simulink model "All_64_Coils"  (R2021b) (continued)
== matlab function All_64_Coils/Coil-3/Outer_5_Coils/Square-10/Square - 10 == (continued)

F_xx_10 = vol_cy * Magentization* (dBxp_1_dx + dBxn_1_dx)/m0;
F_xz_10 = vol_cy * Magentization* (dBxp_1_dz + dBxn_1_dz)/m0;
F_yy_10 = vol_cy * Magentization* (dByp_1_dy + dByn_1_dy)/m0;
F_yz_10 = vol_cy * Magentization* (dByp_1_dz + dByn_1_dz)/m0;
end

== matlab function All_64_Coils/Coil-26/Outer_5_Coils/Square-9/Square - 9 ==
  data: x input; F_xx_9 output; F_xz_9 output; y input; z input; I input; F_yy_9 output; F_yz_9 output
function [F_xx_9, F_xz_9, F_yy_9, F_yz_9] = force(x, y, z, I)

g = 200e-6;     %Coil spacing
m0 = 4*pi*1e-7;
radius = 3e-3;%m
height = 1e-3;%m
Magentization = 1.45; %T
vol_cy = pi*radius^2*height; %If Object is Cylinder, vol in m^3
% vol_sp = (4/3)*(pi * radius^3); %If Object is Sphere, vol in m^3

k = 0.4e-3; %additional length added to wires, to get concentric coil
%--------%--------%--------%--------%--------%--------%--------%----------
% Line along y - axis
%-%--------%--------%--------%--------%--------%--------%--------%--------
y1_1 = -0.6e-3 - 8*k;
y2_1 = 0.6e-3 + 8*k;
a_xp_1 = 9*g;  
a_xn_1 = -9*g;

k_yp_1 = 1/(4*pi*((a_xp_1-x)^2+z^2));
k_1yp_1 = (y2_1-y)/((y2_1-y)^2+(a_xp_1-x)^2+z^2)^0.5;
k_2yp_1 = (y1_1-y)/((y1_1-y)^2+(a_xp_1-x)^2+z^2)^0.5;

k_1yp_3 = (y2_1-y)/((y2_1-y)^2+(a_xp_1-x)^2+z^2)^1.5;
k_2yp_3 = (y1_1-y)/((y1_1-y)^2+(a_xp_1-x)^2+z^2)^1.5;

k_yn_1 = 1/(4*pi*((a_xn_1-x)^2+z^2));
k_1yn_1 = (y2_1-y)/((y2_1-y)^2+(a_xn_1-x)^2+z^2)^0.5;
k_2yn_1 = (y1_1-y)/((y1_1-y)^2+(a_xn_1-x)^2+z^2)^0.5;

k_1yn_3 = (y2_1-y)/((y2_1-y)^2+(a_xn_1-x)^2+z^2)^1.5;
k_2yn_3 = (y1_1-y)/((y1_1-y)^2+(a_xn_1-x)^2+z^2)^1.5;

hyp_x_1 = -(z*k_yp_1*(a_xp_1-x))*I*m0;
hyp_x1_1 = (k_yp_1*(k_1yp_1 - k_2yp_1))*2*4*pi;
hyp_x2_1 = k_1yp_3 - k_2yp_3;
dBxp_1_dx = hyp_x_1*(hyp_x1_1 + hyp_x2_1);

hyp_z_1 = (k_yp_1)*I*m0;
hyp_z1_1 = (k_1yp_1 - k_2yp_1)*(-1);
hyp_z2_1 = (k_yp_1*(k_1yp_1 - k_2yp_1)*(z^2))*4*pi*2;
hyp_z3_1 = (k_1yp_3 - k_2yp_3)*(z^2);
dBxp_1_dz = hyp_z_1*(hyp_z1_1 + hyp_z2_1 + hyp_z3_1);

hyn_x_1 = -(z*k_yn_1*(a_xn_1-x))*I*m0*(-1);
hyn_x1_1 = (k_yn_1*(k_1yn_1 - k_2yn_1))*2*4*pi;
hyn_x2_1 = k_1yn_3 - k_2yn_3;
dBxn_1_dx = hyn_x_1*(hyn_x1_1 + hyn_x2_1);

hyn_z_1 = (k_yn_1)*I*m0*(-1);
hyn_z1_1 = (k_1yn_1 - k_2yn_1)*(-1);
hyn_z2_1 = (k_yn_1*(k_1yn_1 - k_2yn_1)*(z^2))*4*pi*2;
hyn_z3_1 = (k_1yn_3 - k_2yn_3)*(z^2);
dBxn_1_dz = hyn_z_1*(hyn_z1_1 + hyn_z2_1 + hyn_z3_1);

%--------%--------%--------%--------%--------%--------%--------%----------
% Line along x - axis
%-%--------%--------%--------%--------%a--------%--------%--------%--------
x1_1 = -0.6e-3 - 8*k;
x2_1 = 0.6e-3 + 8*k;
a_yp_1 = 9*g;          %Distance of coil from (0,0)
a_yn_1 = -9*g;

k_xp_1 = 1/(4*pi*((a_yp_1-y)^2+z^2));
k_1xp_1 = (x2_1-x)/((x2_1-x)^2+(a_yp_1-y)^2+z^2)^0.5;
k_2xp_1 = (x1_1-x)/((x1_1-x)^2+(a_yp_1-y)^2+z^2)^0.5;

k_xn_1 = 1/(4*pi*((a_yn_1-y)^2+z^2));
k_1xn_1 = (x2_1-x)/((x2_1-x)^2+(a_yn_1-y)^2+z^2)^0.5;
k_2xn_1 = (x1_1-x)/((x1_1-x)^2+(a_yn_1-y)^2+z^2)^0.5;

k_1xp_3 = (x2_1-x)/((x2_1-x)^2+(a_yp_1-y)^2+z^2)^1.5;
k_2xp_3 = (x1_1-x)/((x1_1-x)^2+(a_yp_1-y)^2+z^2)^1.5;

k_1xn_3 = (x2_1-x)/((x2_1-x)^2+(a_yn_1-y)^2+z^2)^1.5;
k_2xn_3 = (x1_1-x)/((x1_1-x)^2+(a_yn_1-y)^2+z^2)^1.5;

hxp_x_1 = -(z*k_xp_1*(a_yp_1-y))*I*m0;
hxp_x1_1 = (k_xp_1*(k_1xp_1 - k_2xp_1))*2*4*pi;
hxp_x2_1 = k_1xp_3 - k_2xp_3;
dByp_1_dy = hxp_x_1*(hxp_x1_1 + hxp_x2_1);

hxp_z_1 = (k_xp_1)*I*m0;
hxp_z1_1 = (k_1xp_1 - k_2xp_1)*(-1);
hxp_z2_1 = (k_xp_1*(k_1xp_1 - k_2xp_1)*(z^2))*4*pi*2;
hxp_z3_1 = (k_1xp_3 - k_2xp_3)*(z^2);
dByp_1_dz = hxp_z_1*(hxp_z1_1 + hxp_z2_1 + hxp_z3_1);

hxn_x_1 = -(z*k_xn_1*(a_yn_1-y))*I*m0*(-1);
hxn_x1_1 = (k_xn_1*(k_1xn_1 - k_2xn_1))*2*4*pi;
hxn_x2_1 = k_1xn_3 - k_2xn_3;
dByn_1_dy = hxn_x_1*(hxn_x1_1 + hxn_x2_1);

hxn_z_1 = (k_xn_1)*I*m0*(-1);
hxn_z1_1 = (k_1xn_1 - k_2xn_1)*(-1);
hxn_z2_1 = (k_xn_1*(k_1xn_1 - k_2xn_1)*(z^2))*4*pi*2;
hxn_z3_1 = (k_1xn_3 - k_2xn_3)*(z^2);
dByn_1_dz = hxn_z_1*(hxn_z1_1 + hxn_z2_1 + hxn_z3_1);

F_xx_9 = vol_cy * Magentization* (dBxp_1_dx + dBxn_1_dx)/m0;
F_xz_9 = vol_cy * Magentization* (dBxp_1_dz + dBxn_1_dz)/m0;
F_yy_9 = vol_cy * Magentization* (dByp_1_dy + dByn_1_dy)/m0;
F_yz_9 = vol_cy * Magentization* (dByp_1_dz + dByn_1_dz)/m0;
end

== matlab function All_64_Coils/Coil-27/Inner_5_coils/Square-1/Square - 1 ==
  data: x input; F_xx_1 output; F_xz_1 output; y input; z input; I input; F_yy_1 output; F_yz_1 output
function [F_xx_1, F_xz_1, F_yy_1, F_yz_1] = force(x, y, z, I)

g = 200e-6;     %Coil spacing
m0 = 4*pi*1e-7;
radius = 3e-3;%m
height = 1e-3;%m
Magentization = 1.45; %T
vol_cy = pi*radius^2*height; %If Object is Cylinder, vol in m^3
% vol_sp = (4/3)*(pi * radius^3); %If Object is Sphere, vol in m^3

k = 0.4e-3; %additional length added to wires, to get concentric coil
%--------%--------%--------%--------%--------%--------%--------%----------
% Line along y - axis
%-%--------%--------%--------%--------%--------%--------%--------%--------
y1_1 = -0.6e-3;
y2_1 = 0.6e-3;
a_xp_1 = g;  
a_xn_1 = -g;

k_yp_1 = 1/(4*pi*((a_xp_1-x)^2+z^2));
k_1yp_1 = (y2_1-y)/((y2_1-y)^2+(a_xp_1-x)^2+z^2)^0.5;
k_2yp_1 = (y1_1-y)/((y1_1-y)^2+(a_xp_1-x)^2+z^2)^0.5;

k_1yp_3 = (y2_1-y)/((y2_1-y)^2+(a_xp_1-x)^2+z^2)^1.5;
k_2yp_3 = (y1_1-y)/((y1_1-y)^2+(a_xp_1-x)^2+z^2)^1.5;

k_yn_1 = 1/(4*pi*((a_xn_1-x)^2+z^2));
k_1yn_1 = (y2_1-y)/((y2_1-y)^2+(a_xn_1-x)^2+z^2)^0.5;
k_2yn_1 = (y1_1-y)/((y1_1-y)^2+(a_xn_1-x)^2+z^2)^0.5;

k_1yn_3 = (y2_1-y)/((y2_1-y)^2+(a_xn_1-x)^2+z^2)^1.5;
k_2yn_3 = (y1_1-y)/((y1_1-y)^2+(a_xn_1-x)^2+z^2)^1.5;

hyp_x_1 = -(z*k_yp_1*(a_xp_1-x))*I*m0;
hyp_x1_1 = (k_yp_1*(k_1yp_1 - k_2yp_1))*2*4*pi;
hyp_x2_1 = k_1yp_3 - k_2yp_3;
dBxp_1_dx = hyp_x_1*(hyp_x1_1 + hyp_x2_1);

hyp_z_1 = (k_yp_1)*I*m0;
hyp_z1_1 = (k_1yp_1 - k_2yp_1)*(-1);
hyp_z2_1 = (k_yp_1*(k_1yp_1 - k_2yp_1)*(z^2))*4*pi*2;
hyp_z3_1 = (k_1yp_3 - k_2yp_3)*(z^2);
dBxp_1_dz = hyp_z_1*(hyp_z1_1 + hyp_z2_1 + hyp_z3_1);

hyn_x_1 = -(z*k_yn_1*(a_xn_1-x))*I*m0*(-1);
hyn_x1_1 = (k_yn_1*(k_1yn_1 - k_2yn_1))*2*4*pi;
hyn_x2_1 = k_1yn_3 - k_2yn_3;
dBxn_1_dx = hyn_x_1*(hyn_x1_1 + hyn_x2_1);

hyn_z_1 = (k_yn_1)*I*m0*(-1);
hyn_z1_1 = (k_1yn_1 - k_2yn_1)*(-1);
hyn_z2_1 = (k_yn_1*(k_1yn_1 - k_2yn_1)*(z^2))*4*pi*2;
hyn_z3_1 = (k_1yn_3 - k_2yn_3)*(z^2);
dBxn_1_dz = hyn_z_1*(hyn_z1_1 + hyn_z2_1 + hyn_z3_1);

%--------%--------%--------%--------%--------%--------%--------%----------
% Line along x - axis
%-%--------%--------%--------%--------%a--------%--------%--------%--------
x1_1 = -0.6e-3;
x2_1 = 0.6e-3;
a_yp_1 = g;          %Distance of coil from (0,0)
a_yn_1 = -g;

k_xp_1 = 1/(4*pi*((a_yp_1-y)^2+z^2));
k_1xp_1 = (x2_1-x)/((x2_1-x)^2+(a_yp_1-y)^2+z^2)^0.5;
k_2xp_1 = (x1_1-x)/((x1_1-x)^2+(a_yp_1-y)^2+z^2)^0.5;

k_xn_1 = 1/(4*pi*((a_yn_1-y)^2+z^2));
k_1xn_1 = (x2_1-x)/((x2_1-x)^2+(a_yn_1-y)^2+z^2)^0.5;
k_2xn_1 = (x1_1-x)/((x1_1-x)^2+(a_yn_1-y)^2+z^2)^0.5;

k_1xp_3 = (x2_1-x)/((x2_1-x)^2+(a_yp_1-y)^2+z^2)^1.5;
k_2xp_3 = (x1_1-x)/((x1_1-x)^2+(a_yp_1-y)^2+z^2)^1.5;

k_1xn_3 = (x2_1-x)/((x2_1-x)^2+(a_yn_1-y)^2+z^2)^1.5;
k_2xn_3 = (x1_1-x)/((x1_1-x)^2+(a_yn_1-y)^2+z^2)^1.5;

hxp_x_1 = -(z*k_xp_1*(a_yp_1-y))*I*m0;
hxp_x1_1 = (k_xp_1*(k_1xp_1 - k_2xp_1))*2*4*pi;
hxp_x2_1 = k_1xp_3 - k_2xp_3;
dByp_1_dy = hxp_x_1*(hxp_x1_1 + hxp_x2_1);

hxp_z_1 = (k_xp_1)*I*m0;
hxp_z1_1 = (k_1xp_1 - k_2xp_1)*(-1);
hxp_z2_1 = (k_xp_1*(k_1xp_1 - k_2xp_1)*(z^2))*4*pi*2;
hxp_z3_1 = (k_1xp_3 - k_2xp_3)*(z^2);
dByp_1_dz = hxp_z_1*(hxp_z1_1 + hxp_z2_1 + hxp_z3_1);

hxn_x_1 = -(z*k_xn_1*(a_yn_1-y))*I*m0*(-1);
hxn_x1_1 = (k_xn_1*(k_1xn_1 - k_2xn_1))*2*4*pi;
hxn_x2_1 = k_1xn_3 - k_2xn_3;
dByn_1_dy = hxn_x_1*(hxn_x1_1 + hxn_x2_1);

hxn_z_1 = (k_xn_1)*I*m0*(-1);
hxn_z1_1 = (k_1xn_1 - k_2xn_1)*(-1);
hxn_z2_1 = (k_xn_1*(k_1xn_1 - k_2xn_1)*(z^2))*4*pi*2;
hxn_z3_1 = (k_1xn_3 - k_2xn_3)*(z^2);
dByn_1_dz = hxn_z_1*(hxn_z1_1 + hxn_z2_1 + hxn_z3_1);

F_xx_1 = vol_cy * Magentization* (dBxp_1_dx + dBxn_1_dx)/m0;
F_xz_1 = vol_cy * Magentization* (dBxp_1_dz + dBxn_1_dz)/m0;
F_yy_1 = vol_cy * Magentization* (dByp_1_dy + dByn_1_dy)/m0;
F_yz_1 = vol_cy * Magentization* (dByp_1_dz + dByn_1_dz)/m0;
end

== matlab function All_64_Coils/Coil-27/Inner_5_coils/Square-2/Square - 2 ==
  data: x input; F_xx_2 output; F_xz_2 output; y input; z input; I input; F_yy_2 output; F_yz_2 output
function [F_xx_2, F_xz_2, F_yy_2, F_yz_2] = force(x, y, z, I)

g = 200e-6;     %Coil spacing
m0 = 4*pi*1e-7;
radius = 3e-3;%m
height = 1e-3;%m
Magentization = 1.45; %T
vol_cy = pi*radius^2*height; %If Object is Cylinder, vol in m^3
% vol_sp = (4/3)*(pi * radius^3); %If Object is Sphere, vol in m^3

k = 0.4e-3; %additional length added to wires, to get concentric coil
%--------%--------%--------%--------%--------%--------%--------%----------
% Line along y - axis
%-%--------%--------%--------%--------%--------%--------%--------%--------
y1_1 = -0.6e-3 - k;
y2_1 = 0.6e-3 + k;
a_xp_1 = 2*g;  
a_xn_1 = -2*g;

k_yp_1 = 1/(4*pi*((a_xp_1-x)^2+z^2));
k_1yp_1 = (y2_1-y)/((y2_1-y)^2+(a_xp_1-x)^2+z^2)^0.5;
k_2yp_1 = (y1_1-y)/((y1_1-y)^2+(a_xp_1-x)^2+z^2)^0.5;

k_1yp_3 = (y2_1-y)/((y2_1-y)^2+(a_xp_1-x)^2+z^2)^1.5;
k_2yp_3 = (y1_1-y)/((y1_1-y)^2+(a_xp_1-x)^2+z^2)^1.5;

k_yn_1 = 1/(4*pi*((a_xn_1-x)^2+z^2));
k_1yn_1 = (y2_1-y)/((y2_1-y)^2+(a_xn_1-x)^2+z^2)^0.5;
k_2yn_1 = (y1_1-y)/((y1_1-y)^2+(a_xn_1-x)^2+z^2)^0.5;

k_1yn_3 = (y2_1-y)/((y2_1-y)^2+(a_xn_1-x)^2+z^2)^1.5;
k_2yn_3 = (y1_1-y)/((y1_1-y)^2+(a_xn_1-x)^2+z^2)^1.5;

hyp_x_1 = -(z*k_yp_1*(a_xp_1-x))*I*m0;
hyp_x1_1 = (k_yp_1*(k_1yp_1 - k_2yp_1))*2*4*pi;
hyp_x2_1 = k_1yp_3 - k_2yp_3;
dBxp_1_dx = hyp_x_1*(hyp_x1_1 + hyp_x2_1);

hyp_z_1 = (k_yp_1)*I*m0;
hyp_z1_1 = (k_1yp_1 - k_2yp_1)*(-1);
hyp_z2_1 = (k_yp_1*(k_1yp_1 - k_2yp_1)*(z^2))*4*pi*2;
hyp_z3_1 = (k_1yp_3 - k_2yp_3)*(z^2);
dBxp_1_dz = hyp_z_1*(hyp_z1_1 + hyp_z2_1 + hyp_z3_1);

hyn_x_1 = -(z*k_yn_1*(a_xn_1-x))*I*m0*(-1);
hyn_x1_1 = (k_yn_1*(k_1yn_1 - k_2yn_1))*2*4*pi;
hyn_x2_1 = k_1yn_3 - k_2yn_3;
dBxn_1_dx = hyn_x_1*(hyn_x1_1 + hyn_x2_1);

hyn_z_1 = (k_yn_1)*I*m0*(-1);
hyn_z1_1 = (k_1yn_1 - k_2yn_1)*(-1);
hyn_z2_1 = (k_yn_1*(k_1yn_1 - k_2yn_1)*(z^2))*4*pi*2;
hyn_z3_1 = (k_1yn_3 - k_2yn_3)*(z^2);
dBxn_1_dz = hyn_z_1*(hyn_z1_1 + hyn_z2_1 + hyn_z3_1);

%--------%--------%--------%--------%--------%--------%--------%----------
% Line along x - axis
%-%--------%--------%--------%--------%a--------%--------%--------%--------
x1_1 = -0.6e-3 - k;
x2_1 = 0.6e-3 + k;
a_yp_1 = 2*g;          %Distance of coil from (0,0)
a_yn_1 = -2*g;

k_xp_1 = 1/(4*pi*((a_yp_1-y)^2+z^2));
k_1xp_1 = (x2_1-x)/((x2_1-x)^2+(a_yp_1-y)^2+z^2)^0.5;
k_2xp_1 = (x1_1-x)/((x1_1-x)^2+(a_yp_1-y)^2+z^2)^0.5;

k_xn_1 = 1/(4*pi*((a_yn_1-y)^2+z^2));
k_1xn_1 = (x2_1-x)/((x2_1-x)^2+(a_yn_1-y)^2+z^2)^0.5;
k_2xn_1 = (x1_1-x)/((x1_1-x)^2+(a_yn_1-y)^2+z^2)^0.5;

k_1xp_3 = (x2_1-x)/((x2_1-x)^2+(a_yp_1-y)^2+z^2)^1.5;
k_2xp_3 = (x1_1-x)/((x1_1-x)^2+(a_yp_1-y)^2+z^2)^1.5;

k_1xn_3 = (x2_1-x)/((x2_1-x)^2+(a_yn_1-y)^2+z^2)^1.5;
k_2xn_3 = (x1_1-x)/((x1_1-x)^2+(a_yn_1-y)^2+z^2)^1.5;

hxp_x_1 = -(z*k_xp_1*(a_yp_1-y))*I*m0;
hxp_x1_1 = (k_xp_1*(k_1xp_1 - k_2xp_1))*2*4*pi;
hxp_x2_1 = k_1xp_3 - k_2xp_3;
dByp_1_dy = hxp_x_1*(hxp_x1_1 + hxp_x2_1);

hxp_z_1 = (k_xp_1)*I*m0;
hxp_z1_1 = (k_1xp_1 - k_2xp_1)*(-1);
hxp_z2_1 = (k_xp_1*(k_1xp_1 - k_2xp_1)*(z^2))*4*pi*2;
hxp_z3_1 = (k_1xp_3 - k_2xp_3)*(z^2);
dByp_1_dz = hxp_z_1*(hxp_z1_1 + hxp_z2_1 + hxp_z3_1);

hxn_x_1 = -(z*k_xn_1*(a_yn_1-y))*I*m0*(-1);
hxn_x1_1 = (k_xn_1*(k_1xn_1 - k_2xn_1))*2*4*pi;
hxn_x2_1 = k_1xn_3 - k_2xn_3;
dByn_1_dy = hxn_x_1*(hxn_x1_1 + hxn_x2_1);

hxn_z_1 = (k_xn_1)*I*m0*(-1);
hxn_z1_1 = (k_1xn_1 - k_2xn_1)*(-1);
hxn_z2_1 = (k_xn_1*(k_1xn_1 - k_2xn_1)*(z^2))*4*pi*2;
hxn_z3_1 = (k_1xn_3 - k_2xn_3)*(z^2);
dByn_1_dz = hxn_z_1*(hxn_z1_1 + hxn_z2_1 + hxn_z3_1);

F_xx_2 = vol_cy * Magentization* (dBxp_1_dx + dBxn_1_dx)/m0;
F_xz_2 = vol_cy * Magentization* (dBxp_1_dz + dBxn_1_dz)/m0;
F_yy_2 = vol_cy * Magentization* (dByp_1_dy + dByn_1_dy)/m0;
F_yz_2 = vol_cy * Magentization* (dByp_1_dz + dByn_1_dz)/m0;
end

== matlab function All_64_Coils/Coil-27/Inner_5_coils/Square-3/Square - 3 ==
  data: x input; F_xx_3 output; F_xz_3 output; y input; z input; I input; F_yy_3 output; F_yz_3 output
function [F_xx_3, F_xz_3, F_yy_3, F_yz_3] = force(x, y, z, I)

g = 200e-6;     %Coil spacing
m0 = 4*pi*1e-7;
radius = 3e-3;%m
height = 1e-3;%m
Magentization = 1.45; %T
vol_cy = pi*radius^2*height; %If Object is Cylinder, vol in m^3
% vol_sp = (4/3)*(pi * radius^3); %If Object is Sphere, vol in m^3

k = 0.4e-3; %additional length added to wires, to get concentric coil
%--------%--------%--------%--------%--------%--------%--------%----------
% Line along y - axis
%-%--------%--------%--------%--------%--------%--------%--------%--------
y1_1 = -0.6e-3 - 2*k;
y2_1 = 0.6e-3 + 2*k;
a_xp_1 = 3*g;  
a_xn_1 = -3*g;

k_yp_1 = 1/(4*pi*((a_xp_1-x)^2+z^2));
k_1yp_1 = (y2_1-y)/((y2_1-y)^2+(a_xp_1-x)^2+z^2)^0.5;
k_2yp_1 = (y1_1-y)/((y1_1-y)^2+(a_xp_1-x)^2+z^2)^0.5;

k_1yp_3 = (y2_1-y)/((y2_1-y)^2+(a_xp_1-x)^2+z^2)^1.5;
k_2yp_3 = (y1_1-y)/((y1_1-y)^2+(a_xp_1-x)^2+z^2)^1.5;

k_yn_1 = 1/(4*pi*((a_xn_1-x)^2+z^2));
k_1yn_1 = (y2_1-y)/((y2_1-y)^2+(a_xn_1-x)^2+z^2)^0.5;
k_2yn_1 = (y1_1-y)/((y1_1-y)^2+(a_xn_1-x)^2+z^2)^0.5;

k_1yn_3 = (y2_1-y)/((y2_1-y)^2+(a_xn_1-x)^2+z^2)^1.5;
k_2yn_3 = (y1_1-y)/((y1_1-y)^2+(a_xn_1-x)^2+z^2)^1.5;

hyp_x_1 = -(z*k_yp_1*(a_xp_1-x))*I*m0;
hyp_x1_1 = (k_yp_1*(k_1yp_1 - k_2yp_1))*2*4*pi;
hyp_x2_1 = k_1yp_3 - k_2yp_3;
dBxp_1_dx = hyp_x_1*(hyp_x1_1 + hyp_x2_1);

hyp_z_1 = (k_yp_1)*I*m0;
hyp_z1_1 = (k_1yp_1 - k_2yp_1)*(-1);
hyp_z2_1 = (k_yp_1*(k_1yp_1 - k_2yp_1)*(z^2))*4*pi*2;
hyp_z3_1 = (k_1yp_3 - k_2yp_3)*(z^2);
dBxp_1_dz = hyp_z_1*(hyp_z1_1 + hyp_z2_1 + hyp_z3_1);

hyn_x_1 = -(z*k_yn_1*(a_xn_1-x))*I*m0*(-1);
hyn_x1_1 = (k_yn_1*(k_1yn_1 - k_2yn_1))*2*4*pi;
hyn_x2_1 = k_1yn_3 - k_2yn_3;
dBxn_1_dx = hyn_x_1*(hyn_x1_1 + hyn_x2_1);

hyn_z_1 = (k_yn_1)*I*m0*(-1);
hyn_z1_1 = (k_1yn_1 - k_2yn_1)*(-1);
hyn_z2_1 = (k_yn_1*(k_1yn_1 - k_2yn_1)*(z^2))*4*pi*2;
hyn_z3_1 = (k_1yn_3 - k_2yn_3)*(z^2);
dBxn_1_dz = hyn_z_1*(hyn_z1_1 + hyn_z2_1 + hyn_z3_1);

%--------%--------%--------%--------%--------%--------%--------%----------
% Line along x - axis
%-%--------%--------%--------%--------%a--------%--------%--------%--------
x1_1 = -0.6e-3 - 2*k;
x2_1 = 0.6e-3 + 2*k;
a_yp_1 = 3*g;          %Distance of coil from (0,0)
a_yn_1 = -3*g;

k_xp_1 = 1/(4*pi*((a_yp_1-y)^2+z^2));
k_1xp_1 = (x2_1-x)/((x2_1-x)^2+(a_yp_1-y)^2+z^2)^0.5;
k_2xp_1 = (x1_1-x)/((x1_1-x)^2+(a_yp_1-y)^2+z^2)^0.5;

k_xn_1 = 1/(4*pi*((a_yn_1-y)^2+z^2));
k_1xn_1 = (x2_1-x)/((x2_1-x)^2+(a_yn_1-y)^2+z^2)^0.5;
k_2xn_1 = (x1_1-x)/((x1_1-x)^2+(a_yn_1-y)^2+z^2)^0.5;

k_1xp_3 = (x2_1-x)/((x2_1-x)^2+(a_yp_1-y)^2+z^2)^1.5;
k_2xp_3 = (x1_1-x)/((x1_1-x)^2+(a_yp_1-y)^2+z^2)^1.5;

k_1xn_3 = (x2_1-x)/((x2_1-x)^2+(a_yn_1-y)^2+z^2)^1.5;
k_2xn_3 = (x1_1-x)/((x1_1-x)^2+(a_yn_1-y)^2+z^2)^1.5;

hxp_x_1 = -(z*k_xp_1*(a_yp_1-y))*I*m0;
hxp_x1_1 = (k_xp_1*(k_1xp_1 - k_2xp_1))*2*4*pi;
hxp_x2_1 = k_1xp_3 - k_2xp_3;
dByp_1_dy = hxp_x_1*(hxp_x1_1 + hxp_x2_1);

hxp_z_1 = (k_xp_1)*I*m0;
hxp_z1_1 = (k_1xp_1 - k_2xp_1)*(-1);
hxp_z2_1 = (k_xp_1*(k_1xp_1 - k_2xp_1)*(z^2))*4*pi*2;
hxp_z3_1 = (k_1xp_3 - k_2xp_3)*(z^2);
dByp_1_dz = hxp_z_1*(hxp_z1_1 + hxp_z2_1 + hxp_z3_1);

hxn_x_1 = -(z*k_xn_1*(a_yn_1-y))*I*m0*(-1);
hxn_x1_1 = (k_xn_1*(k_1xn_1 - k_2xn_1))*2*4*pi;
hxn_x2_1 = k_1xn_3 - k_2xn_3;
dByn_1_dy = hxn_x_1*(hxn_x1_1 + hxn_x2_1);

hxn_z_1 = (k_xn_1)*I*m0*(-1);
hxn_z1_1 = (k_1xn_1 - k_2xn_1)*(-1);
hxn_z2_1 = (k_xn_1*(k_1xn_1 - k_2xn_1)*(z^2))*4*pi*2;
hxn_z3_1 = (k_1xn_3 - k_2xn_3)*(z^2);
dByn_1_dz = hxn_z_1*(hxn_z1_1 + hxn_z2_1 + hxn_z3_1);

F_xx_3 = vol_cy * Magentization* (dBxp_1_dx + dBxn_1_dx)/m0;
F_xz_3 = vol_cy * Magentization* (dBxp_1_dz + dBxn_1_dz)/m0;
F_yy_3 = vol_cy * Magentization* (dByp_1_dy + dByn_1_dy)/m0;
F_yz_3 = vol_cy * Magentization* (dByp_1_dz + dByn_1_dz)/m0;
end

== matlab function All_64_Coils/Coil-27/Inner_5_coils/Square-4/Square - 4 ==
  data: x input; F_xx_4 output; F_xz_4 output; y input; z input; I input; F_yy_4 output; F_yz_4 output
function [F_xx_4, F_xz_4, F_yy_4, F_yz_4] = force(x, y, z, I)

g = 200e-6;     %Coil spacing
m0 = 4*pi*1e-7;
radius = 3e-3;%m
height = 1e-3;%m
Magentization = 1.45; %T
vol_cy = pi*radius^2*height; %If Object is Cylinder, vol in m^3
% vol_sp = (4/3)*(pi * radius^3); %If Object is Sphere, vol in m^3

k = 0.4e-3; %additional length added to wires, to get concentric coil
%--------%--------%--------%--------%--------%--------%--------%----------
% Line along y - axis
%-%--------%--------%--------%--------%--------%--------%--------%--------
y1_1 = -0.6e-3 - 3*k;
y2_1 = 0.6e-3 + 3*k;
a_xp_1 = 4*g;  
a_xn_1 = -4*g;

k_yp_1 = 1/(4*pi*((a_xp_1-x)^2+z^2));
k_1yp_1 = (y2_1-y)/((y2_1-y)^2+(a_xp_1-x)^2+z^2)^0.5;
k_2yp_1 = (y1_1-y)/((y1_1-y)^2+(a_xp_1-x)^2+z^2)^0.5;

k_1yp_3 = (y2_1-y)/((y2_1-y)^2+(a_xp_1-x)^2+z^2)^1.5;
k_2yp_3 = (y1_1-y)/((y1_1-y)^2+(a_xp_1-x)^2+z^2)^1.5;

k_yn_1 = 1/(4*pi*((a_xn_1-x)^2+z^2));
k_1yn_1 = (y2_1-y)/((y2_1-y)^2+(a_xn_1-x)^2+z^2)^0.5;
k_2yn_1 = (y1_1-y)/((y1_1-y)^2+(a_xn_1-x)^2+z^2)^0.5;

k_1yn_3 = (y2_1-y)/((y2_1-y)^2+(a_xn_1-x)^2+z^2)^1.5;
k_2yn_3 = (y1_1-y)/((y1_1-y)^2+(a_xn_1-x)^2+z^2)^1.5;

hyp_x_1 = -(z*k_yp_1*(a_xp_1-x))*I*m0;
hyp_x1_1 = (k_yp_1*(k_1yp_1 - k_2yp_1))*2*4*pi;
hyp_x2_1 = k_1yp_3 - k_2yp_3;
dBxp_1_dx = hyp_x_1*(hyp_x1_1 + hyp_x2_1);

hyp_z_1 = (k_yp_1)*I*m0;
hyp_z1_1 = (k_1yp_1 - k_2yp_1)*(-1);
hyp_z2_1 = (k_yp_1*(k_1yp_1 - k_2yp_1)*(z^2))*4*pi*2;
hyp_z3_1 = (k_1yp_3 - k_2yp_3)*(z^2);
dBxp_1_dz = hyp_z_1*(hyp_z1_1 + hyp_z2_1 + hyp_z3_1);

hyn_x_1 = -(z*k_yn_1*(a_xn_1-x))*I*m0*(-1);
hyn_x1_1 = (k_yn_1*(k_1yn_1 - k_2yn_1))*2*4*pi;
hyn_x2_1 = k_1yn_3 - k_2yn_3;
dBxn_1_dx = hyn_x_1*(hyn_x1_1 + hyn_x2_1);

hyn_z_1 = (k_yn_1)*I*m0*(-1);
hyn_z1_1 = (k_1yn_1 - k_2yn_1)*(-1);
hyn_z2_1 = (k_yn_1*(k_1yn_1 - k_2yn_1)*(z^2))*4*pi*2;
hyn_z3_1 = (k_1yn_3 - k_2yn_3)*(z^2);
dBxn_1_dz = hyn_z_1*(hyn_z1_1 + hyn_z2_1 + hyn_z3_1);

%--------%--------%--------%--------%--------%--------%--------%----------
% Line along x - axis
%-%--------%--------%--------%--------%a--------%--------%--------%--------
x1_1 = -0.6e-3 - 3*k;
x2_1 = 0.6e-3 + 3*k;
a_yp_1 = 4*g;          %Distance of coil from (0,0)
a_yn_1 = -4*g;

k_xp_1 = 1/(4*pi*((a_yp_1-y)^2+z^2));
k_1xp_1 = (x2_1-x)/((x2_1-x)^2+(a_yp_1-y)^2+z^2)^0.5;
k_2xp_1 = (x1_1-x)/((x1_1-x)^2+(a_yp_1-y)^2+z^2)^0.5;

k_xn_1 = 1/(4*pi*((a_yn_1-y)^2+z^2));
k_1xn_1 = (x2_1-x)/((x2_1-x)^2+(a_yn_1-y)^2+z^2)^0.5;
k_2xn_1 = (x1_1-x)/((x1_1-x)^2+(a_yn_1-y)^2+z^2)^0.5;

k_1xp_3 = (x2_1-x)/((x2_1-x)^2+(a_yp_1-y)^2+z^2)^1.5;
k_2xp_3 = (x1_1-x)/((x1_1-x)^2+(a_yp_1-y)^2+z^2)^1.5;

k_1xn_3 = (x2_1-x)/((x2_1-x)^2+(a_yn_1-y)^2+z^2)^1.5;
k_2xn_3 = (x1_1-x)/((x1_1-x)^2+(a_yn_1-y)^2+z^2)^1.5;

hxp_x_1 = -(z*k_xp_1*(a_yp_1-y))*I*m0;
hxp_x1_1 = (k_xp_1*(k_1xp_1 - k_2xp_1))*2*4*pi;
hxp_x2_1 = k_1xp_3 - k_2xp_3;
dByp_1_dy = hxp_x_1*(hxp_x1_1 + hxp_x2_1);

hxp_z_1 = (k_xp_1)*I*m0;
hxp_z1_1 = (k_1xp_1 - k_2xp_1)*(-1);
hxp_z2_1 = (k_xp_1*(k_1xp_1 - k_2xp_1)*(z^2))*4*pi*2;
hxp_z3_1 = (k_1xp_3 - k_2xp_3)*(z^2);
dByp_1_dz = hxp_z_1*(hxp_z1_1 + hxp_z2_1 + hxp_z3_1);

hxn_x_1 = -(z*k_xn_1*(a_yn_1-y))*I*m0*(-1);
hxn_x1_1 = (k_xn_1*(k_1xn_1 - k_2xn_1))*2*4*pi;
hxn_x2_1 = k_1xn_3 - k_2xn_3;
dByn_1_dy = hxn_x_1*(hxn_x1_1 + hxn_x2_1);

hxn_z_1 = (k_xn_1)*I*m0*(-1);
hxn_z1_1 = (k_1xn_1 - k_2xn_1)*(-1);
hxn_z2_1 = (k_xn_1*(k_1xn_1 - k_2xn_1)*(z^2))*4*pi*2;
hxn_z3_1 = (k_1xn_3 - k_2xn_3)*(z^2);
dByn_1_dz = hxn_z_1*(hxn_z1_1 + hxn_z2_1 + hxn_z3_1);

F_xx_4 = vol_cy * Magentization* (dBxp_1_dx + dBxn_1_dx)/m0;
F_xz_4 = vol_cy * Magentization* (dBxp_1_dz + dBxn_1_dz)/m0;
F_yy_4 = vol_cy * Magentization* (dByp_1_dy + dByn_1_dy)/m0;
F_yz_4 = vol_cy * Magentization* (dByp_1_dz + dByn_1_dz)/m0;
end

== matlab function All_64_Coils/Coil-27/Inner_5_coils/Square-5/Square - 5 ==
  data: x input; F_xx_5 output; F_xz_5 output; y input; z input; I input; F_yy_5 output; F_yz_5 output
function [F_xx_5, F_xz_5, F_yy_5, F_yz_5] = force(x, y, z, I)

g = 200e-6;     %Coil spacing
m0 = 4*pi*1e-7;
radius = 3e-3;%m
height = 1e-3;%m
Magentization = 1.45; %T
vol_cy = pi*radius^2*height; %If Object is Cylinder, vol in m^3
% vol_sp = (4/3)*(pi * radius^3); %If Object is Sphere, vol in m^3

k = 0.4e-3; %additional length added to wires, to get concentric coil
%--------%--------%--------%--------%--------%--------%--------%----------
% Line along y - axis
%-%--------%--------%--------%--------%--------%--------%--------%--------
y1_1 = -0.6e-3 - 4*k;
y2_1 = 0.6e-3 + 4*k;
a_xp_1 = 5*g;  
a_xn_1 = -5*g;

k_yp_1 = 1/(4*pi*((a_xp_1-x)^2+z^2));
k_1yp_1 = (y2_1-y)/((y2_1-y)^2+(a_xp_1-x)^2+z^2)^0.5;
k_2yp_1 = (y1_1-y)/((y1_1-y)^2+(a_xp_1-x)^2+z^2)^0.5;

k_1yp_3 = (y2_1-y)/((y2_1-y)^2+(a_xp_1-x)^2+z^2)^1.5;
k_2yp_3 = (y1_1-y)/((y1_1-y)^2+(a_xp_1-x)^2+z^2)^1.5;

k_yn_1 = 1/(4*pi*((a_xn_1-x)^2+z^2));
k_1yn_1 = (y2_1-y)/((y2_1-y)^2+(a_xn_1-x)^2+z^2)^0.5;
k_2yn_1 = (y1_1-y)/((y1_1-y)^2+(a_xn_1-x)^2+z^2)^0.5;

k_1yn_3 = (y2_1-y)/((y2_1-y)^2+(a_xn_1-x)^2+z^2)^1.5;
k_2yn_3 = (y1_1-y)/((y1_1-y)^2+(a_xn_1-x)^2+z^2)^1.5;

hyp_x_1 = -(z*k_yp_1*(a_xp_1-x))*I*m0;
hyp_x1_1 = (k_yp_1*(k_1yp_1 - k_2yp_1))*2*4*pi;
hyp_x2_1 = k_1yp_3 - k_2yp_3;
dBxp_1_dx = hyp_x_1*(hyp_x1_1 + hyp_x2_1);

hyp_z_1 = (k_yp_1)*I*m0;
hyp_z1_1 = (k_1yp_1 - k_2yp_1)*(-1);
hyp_z2_1 = (k_yp_1*(k_1yp_1 - k_2yp_1)*(z^2))*4*pi*2;
hyp_z3_1 = (k_1yp_3 - k_2yp_3)*(z^2);
dBxp_1_dz = hyp_z_1*(hyp_z1_1 + hyp_z2_1 + hyp_z3_1);

hyn_x_1 = -(z*k_yn_1*(a_xn_1-x))*I*m0*(-1);
hyn_x1_1 = (k_yn_1*(k_1yn_1 - k_2yn_1))*2*4*pi;
hyn_x2_1 = k_1yn_3 - k_2yn_3;
dBxn_1_dx = hyn_x_1*(hyn_x1_1 + hyn_x2_1);

hyn_z_1 = (k_yn_1)*I*m0*(-1);
hyn_z1_1 = (k_1yn_1 - k_2yn_1)*(-1);
hyn_z2_1 = (k_yn_1*(k_1yn_1 - k_2yn_1)*(z^2))*4*pi*2;
hyn_z3_1 = (k_1yn_3 - k_2yn_3)*(z^2);
dBxn_1_dz = hyn_z_1*(hyn_z1_1 + hyn_z2_1 + hyn_z3_1);

%--------%--------%--------%--------%--------%--------%--------%----------
% Line along x - axis
%-%--------%--------%--------%--------%a--------%--------%--------%--------
x1_1 = -0.6e-3 - 4*k;
x2_1 = 0.6e-3 + 4*k;
a_yp_1 = 5*g;          %Distance of coil from (0,0)
a_yn_1 = -5*g;

k_xp_1 = 1/(4*pi*((a_yp_1-y)^2+z^2));
k_1xp_1 = (x2_1-x)/((x2_1-x)^2+(a_yp_1-y)^2+z^2)^0.5;
k_2xp_1 = (x1_1-x)/((x1_1-x)^2+(a_yp_1-y)^2+z^2)^0.5;

k_xn_1 = 1/(4*pi*((a_yn_1-y)^2+z^2));
k_1xn_1 = (x2_1-x)/((x2_1-x)^2+(a_yn_1-y)^2+z^2)^0.5;
k_2xn_1 = (x1_1-x)/((x1_1-x)^2+(a_yn_1-y)^2+z^2)^0.5;

k_1xp_3 = (x2_1-x)/((x2_1-x)^2+(a_yp_1-y)^2+z^2)^1.5;
k_2xp_3 = (x1_1-x)/((x1_1-x)^2+(a_yp_1-y)^2+z^2)^1.5;

k_1xn_3 = (x2_1-x)/((x2_1-x)^2+(a_yn_1-y)^2+z^2)^1.5;
k_2xn_3 = (x1_1-x)/((x1_1-x)^2+(a_yn_1-y)^2+z^2)^1.5;

hxp_x_1 = -(z*k_xp_1*(a_yp_1-y))*I*m0;
hxp_x1_1 = (k_xp_1*(k_1xp_1 - k_2xp_1))*2*4*pi;
hxp_x2_1 = k_1xp_3 - k_2xp_3;
dByp_1_dy = hxp_x_1*(hxp_x1_1 + hxp_x2_1);

hxp_z_1 = (k_xp_1)*I*m0;
hxp_z1_1 = (k_1xp_1 - k_2xp_1)*(-1);
hxp_z2_1 = (k_xp_1*(k_1xp_1 - k_2xp_1)*(z^2))*4*pi*2;
hxp_z3_1 = (k_1xp_3 - k_2xp_3)*(z^2);
dByp_1_dz = hxp_z_1*(hxp_z1_1 + hxp_z2_1 + hxp_z3_1);

hxn_x_1 = -(z*k_xn_1*(a_yn_1-y))*I*m0*(-1);
hxn_x1_1 = (k_xn_1*(k_1xn_1 - k_2xn_1))*2*4*pi;
hxn_x2_1 = k_1xn_3 - k_2xn_3;
dByn_1_dy = hxn_x_1*(hxn_x1_1 + hxn_x2_1);

hxn_z_1 = (k_xn_1)*I*m0*(-1);
hxn_z1_1 = (k_1xn_1 - k_2xn_1)*(-1);
hxn_z2_1 = (k_xn_1*(k_1xn_1 - k_2xn_1)*(z^2))*4*pi*2;
hxn_z3_1 = (k_1xn_3 - k_2xn_3)*(z^2);
dByn_1_dz = hxn_z_1*(hxn_z1_1 + hxn_z2_1 + hxn_z3_1);

F_xx_5 = vol_cy * Magentization* (dBxp_1_dx + dBxn_1_dx)/m0;
F_xz_5 = vol_cy * Magentization* (dBxp_1_dz + dBxn_1_dz)/m0;
F_yy_5 = vol_cy * Magentization* (dByp_1_dy + dByn_1_dy)/m0;
F_yz_5 = vol_cy * Magentization* (dByp_1_dz + dByn_1_dz)/m0;
end
